simulink model "ClimaRateLimMgr"  (R2022b)
settings: Solver=FixedStepDiscrete  StartTime=0.0  StopTime=10.0  FixedStep=0.2  RelTol=1e-3
(part 1 of 2)
settings Model: Creator=blue.ge  LastModifiedBy=blue.ge
settings DataIOCC: SaveFormat=StructureWithTime
settings OptimizationCC: DefaultParameterBehavior=Inlined  EnhancedBackFolding=on  PassReuseOutputArgsAs=Individual arguments  ZeroExternalMemoryAtStartup=off  ZeroInternalMemoryAtStartup=off  OptimizeBlockOrder=speed  DifferentSizesBufferReuse=on  OptimizationCustomize=off  EfficientTunableParamExpr=on
settings DebuggingCC: ParameterTunabilityLossMsg=error
settings ModelReferenceCC: ModelReferenceNumInstancesAllowed=Single
settings SFSimCC: MATLABDynamicMemAlloc=off
settings RTWCC: Description=AUTOSAR  SystemTargetFile=autosar.tlc  GenCodeOnly=on  TemplateMakefile=ert_default_tmf
settings CodeAppCC: ForceParamTrailComments=on  IgnoreCustomStorageClasses=off  OperatorAnnotations=on  SimulinkDataObjDesc=on  SFDataObjDesc=on  InsertBlockDesc=on
settings STFCustomTargetCC: TargetLangStandard=C99 (ISO)  MultiwordLength=256  ParMdlRefBuildCompliant=on  CompOptLevelCompliant=on  IncludeMdlTerminateFcn=off  CombineOutputUpdateFcns=on  SuppressErrorStatus=on  ERTCustomFileBanners=on  SupportNonFinite=off  SupportComplex=off  SupportContinuousTime=off  SupportNonInlinedSFcns=off  RemoveResetFunc=on  MATLABClassNameForMDSCustomization=Simulink.SoftwareTarget.ERTCustomization  AutosarCompliant=on  UseToolchainInfoCompliant=on  LookupTableObjectStructAxisOrder=2,1,3,4,...  LUTObjectStructOrderEvenSpacing=Size,Table,Breakpoints  SystemTargetFile=autosar.tlc
settings ERTTargetCC: TargetLangStandard=C99 (ISO)  ConcurrentExecutionCompliant=off  IncludeMdlTerminateFcn=off  SuppressErrorStatus=on  SupportNonFinite=off  SupportComplex=off  ModelStepFunctionPrototypeControlCompliant=off  CPPClassGenCompliant=off  AutosarCompliant=on  LookupTableObjectStructAxisOrder=2,1,3,4,...  LUTObjectStructOrderEvenSpacing=Size,Table,Breakpoints  InlinedParameterPlacement=NonHierarchical  ERTCustomFileTemplate=example_file_process.tlc

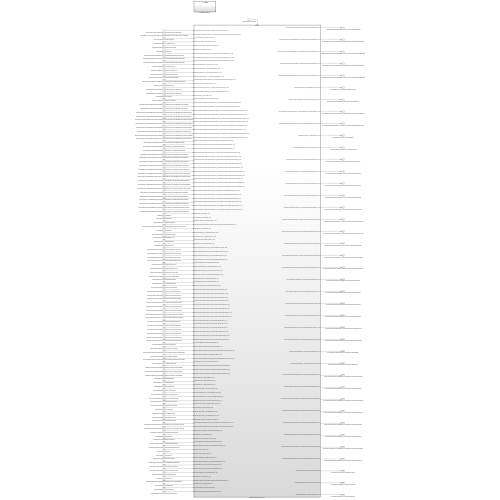
[thumbnail: Simulink's stored preview of the top-level diagram]
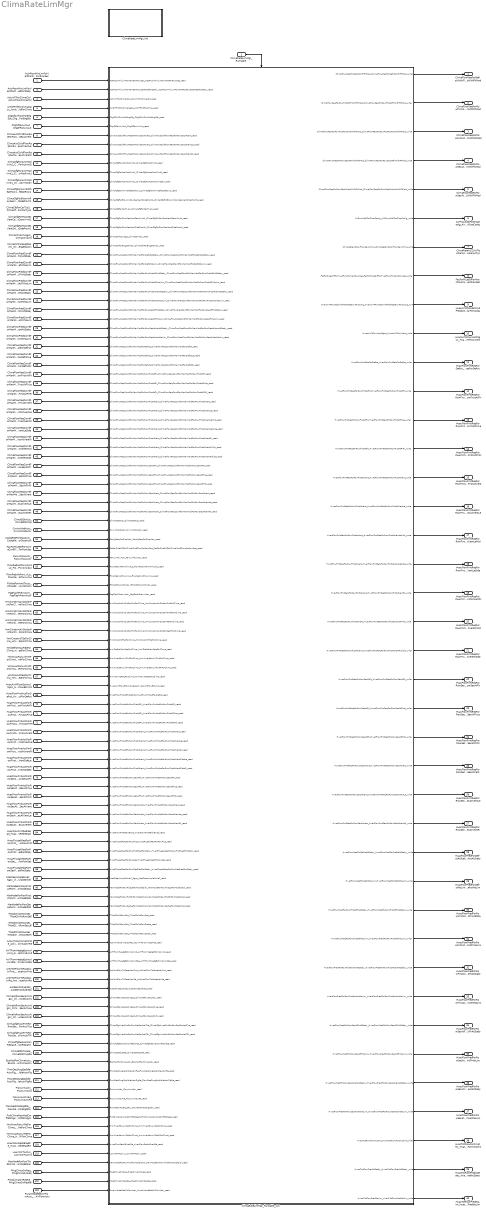
== system ClimaRateLimMgr ==
  ClimaRateLimMgr_Runnable : Inport  SampleTime=0.2  OutputFunctionCall=on
  ActrPosnMinLimForVentForRowSec_ActrPosnMinLimForVentForRowSec : Inport  Port=2  OutDataTypeStr=Perc30  PortDimensions=1
  ActrPosnMinLimForVentForRowSecPredForStaby_ActrPosnMinLimForVentForRowSecPredForStaby : Inport  Port=3  OutDataTypeStr=Perc30  PortDimensions=1
  ActvnTiForClimaCtrl_ActvnTiForClimaCtrl : Inport  Port=4  OutDataTypeStr=Ti33  PortDimensions=1
  AmbTFildForClimaNgcc_AmbTFildForClima : Inport  Port=5  OutDataTypeStr=T30  PortDimensions=1
  ChgOfAirFlowForEngStb_ChgOfAirFlowForEngStb : Inport  Port=6  OutDataTypeStr=Boolean  PortDimensions=1
  ChgOfFanLvlAut_ChgOfFanLvlAut : Inport  Port=7  OutDataTypeStr=Boolean  PortDimensions=1
  ClimaAutCtrldFlowReqForRowSecAtVent_ClimaAutCtrldFlowReqForRowSecAtVent : Inport  Port=8  OutDataTypeStr=AirFlow30  PortDimensions=1
  ClimaAutCtrldFlowReqForRowSecAtVent_ClimaAutCtrldFlowReqForRowSecAtVentLe : Inport  Port=9  OutDataTypeStr=AirFlow30  PortDimensions=1
  ClimaAutCtrldFlowReqForRowSecAtVent_ClimaAutCtrldFlowReqForRowSecAtVentRi : Inport  Port=10  OutDataTypeStr=AirFlow30  PortDimensions=1
  ClimaCfgForActrModVrnt1_ClimaCfgForActrInd1 : Inport  Port=11  OutDataTypeStr=Enum: ClimaCfgForActrInd1  PortDimensions=1
  ClimaCfgForActrModVrnt1_ClimaCfgForActrModVrnt1 : Inport  Port=12  OutDataTypeStr=Enum: ClimaCfgForActrModVrnt1  PortDimensions=1
  ClimaCfgForActrModVrnt1_ClimaCfgForActrModVrntCen : Inport  Port=13  OutDataTypeStr=Enum: ClimaCfgForActrModVrntCen  PortDimensions=1
  ClimaCfgForAmntOfSeatRow1_ClimaCfgForAmntOfSeatRow1 : Inport  Port=14  OutDataTypeStr=Enum: ClimaCfgForAmntOfSeatRow1  PortDimensions=1
  ClimaCfgForBlowrInRowSecVentSidePrsnt1_ClimaCfgForBlowrInRowSecVentSidePrsnt1 : Inport  Port=15  OutDataTypeStr=Boolean  PortDimensions=1
  ClimaCfgForCarTyp1_ClimaCfgForCarTyp1 : Inport  Port=16  OutDataTypeStr=Enum: ClimaCfgForCarTyp1  PortDimensions=1
  ClimaCfgForRowSecVentCentrVrnt1_ClimaCfgForRowSecVentCentrVrnt1 : Inport  Port=17  OutDataTypeStr=Enum: ClimaCfgForRowSecVentCentrVrnt1  PortDimensions=1
  ClimaCfgForRowSecVentSidePrsnt1_ClimaCfgForRowSecVentSidePrsnt1 : Inport  Port=18  OutDataTypeStr=Boolean  PortDimensions=1
  ClimaCtrlActvNgcc_ClimaCtrlActv : Inport  Port=19  OutDataTypeStr=Boolean  PortDimensions=1
  ClimaCtrlForEngStbActv_ClimaCtrlForEngStbActv : Inport  Port=20  OutDataTypeStr=Boolean  PortDimensions=1
  ClimaFlowPredCorrdForManModForDefrstAtStaby_ClimaFlowPredCorrdForManModForDefrstAtStaby : Inport  Port=21  OutDataTypeStr=AirFlow30  PortDimensions=1
  ClimaFlowPredCorrdForManModForDefrstNoLim_ClimaFlowPredCorrdForManModForDefrstNoLim : Inport  Port=22  OutDataTypeStr=AirFlow30  PortDimensions=1
  ClimaFlowPredCorrdForManModForRowFirstAtFlrAtStaby_ClimaFlowPredCorrdForManModForRowFirstAtFlrAtStaby : Inport  Port=23  OutDataTypeStr=AirFlow30  PortDimensions=1
  ClimaFlowPredCorrdForManModForRowFirstAtFlrNoLim_ClimaFlowPredCorrdForManModForRowFirstAtFlrNoLim : Inport  Port=24  OutDataTypeStr=AirFlow30  PortDimensions=1
  ClimaFlowPredCorrdForManModForRowFirstAtVentAtStaby_ClimaFlowPredCorrdForManModForRowFirstAtVentAtStaby : Inport  Port=25  OutDataTypeStr=AirFlow30  PortDimensions=1
  ClimaFlowPredCorrdForManModForRowFirstAtVentNoLim_ClimaFlowPredCorrdForManModForRowFirstAtVentNoLim : Inport  Port=26  OutDataTypeStr=AirFlow30  PortDimensions=1
  ClimaFlowPredCorrdForManModForRowSecAtFlrAtStaby_ClimaFlowPredCorrdForManModForRowSecAtFlrAtStaby : Inport  Port=27  OutDataTypeStr=AirFlow30  PortDimensions=1
  ClimaFlowPredCorrdForManModForRowSecAtFlrNoLim_ClimaFlowPredCorrdForManModForRowSecAtFlrNoLim : Inport  Port=28  OutDataTypeStr=AirFlow30  PortDimensions=1
  ClimaFlowPredCorrdForManModForRowSecAtVentAtStaby_ClimaFlowPredCorrdForManModForRowSecAtVentAtStaby : Inport  Port=29  OutDataTypeStr=AirFlow30  PortDimensions=1
  ClimaFlowPredCorrdForManModForRowSecAtVentNoLim_ClimaFlowPredCorrdForManModForRowSecAtVentNoLim : Inport  Port=30  OutDataTypeStr=AirFlow30  PortDimensions=1
  ClimaFlowReqCorrdForManModForDefrst_ClimaFlowReqCorrdForManModForDefrst : Inport  Port=31  OutDataTypeStr=AirFlow30  PortDimensions=1
  ClimaFlowReqCorrdForManModForDefrst_ClimaFlowReqCorrdForManModForDefrstLe : Inport  Port=32  OutDataTypeStr=AirFlow30  PortDimensions=1
  ClimaFlowReqCorrdForManModForDefrst_ClimaFlowReqCorrdForManModForDefrstRi : Inport  Port=33  OutDataTypeStr=AirFlow30  PortDimensions=1
  ClimaFlowReqCorrdForManModForRowFirstAtFlr_ClimaFlowReqCorrdForManModForRowFirstAtFlr : Inport  Port=34  OutDataTypeStr=AirFlow30  PortDimensions=1
  ClimaFlowReqCorrdForManModForRowFirstAtFlr_ClimaFlowReqCorrdForManModForRowFirstAtFlrLe : Inport  Port=35  OutDataTypeStr=AirFlow30  PortDimensions=1
  ClimaFlowReqCorrdForManModForRowFirstAtFlr_ClimaFlowReqCorrdForManModForRowFirstAtFlrRi : Inport  Port=36  OutDataTypeStr=AirFlow30  PortDimensions=1
  ClimaFlowReqCorrdForManModForRowFirstAtVent_ClimaFlowReqCorrdForManModForRowFirstAtVent : Inport  Port=37  OutDataTypeStr=AirFlow30  PortDimensions=1
  ClimaFlowReqCorrdForManModForRowFirstAtVent_ClimaFlowReqCorrdForManModForRowFirstAtVentLe : Inport  Port=38  OutDataTypeStr=AirFlow30  PortDimensions=1
  ClimaFlowReqCorrdForManModForRowFirstAtVent_ClimaFlowReqCorrdForManModForRowFirstAtVentLeMid : Inport  Port=39  OutDataTypeStr=AirFlow30  PortDimensions=1
  ClimaFlowReqCorrdForManModForRowFirstAtVent_ClimaFlowReqCorrdForManModForRowFirstAtVentLeSide : Inport  Port=40  OutDataTypeStr=AirFlow30  PortDimensions=1
  ClimaFlowReqCorrdForManModForRowFirstAtVent_ClimaFlowReqCorrdForManModForRowFirstAtVentRi : Inport  Port=41  OutDataTypeStr=AirFlow30  PortDimensions=1
  ClimaFlowReqCorrdForManModForRowFirstAtVent_ClimaFlowReqCorrdForManModForRowFirstAtVentRiMid : Inport  Port=42  OutDataTypeStr=AirFlow30  PortDimensions=1
  ClimaFlowReqCorrdForManModForRowFirstAtVent_ClimaFlowReqCorrdForManModForRowFirstAtVentRiSide : Inport  Port=43  OutDataTypeStr=AirFlow30  PortDimensions=1
  ClimaFlowReqCorrdForManModForRowSecAtFlr_ClimaFlowReqCorrdForManModForRowSecAtFlr : Inport  Port=44  OutDataTypeStr=AirFlow30  PortDimensions=1
  ClimaFlowReqCorrdForManModForRowSecAtFlr_ClimaFlowReqCorrdForManModForRowSecAtFlrLe : Inport  Port=45  OutDataTypeStr=AirFlow30  PortDimensions=1
  ClimaFlowReqCorrdForManModForRowSecAtFlr_ClimaFlowReqCorrdForManModForRowSecAtFlrRi : Inport  Port=46  OutDataTypeStr=AirFlow30  PortDimensions=1
  ClimaFlowReqCorrdForManModForRowSecAtVent_ClimaFlowReqCorrdForManModForRowSecAtVent : Inport  Port=47  OutDataTypeStr=AirFlow30  PortDimensions=1
  ClimaFlowReqCorrdForManModForRowSecAtVent_ClimaFlowReqCorrdForManModForRowSecAtVentLe : Inport  Port=48  OutDataTypeStr=AirFlow30  PortDimensions=1
  ClimaFlowReqCorrdForManModForRowSecAtVent_ClimaFlowReqCorrdForManModForRowSecAtVentRi : Inport  Port=49  OutDataTypeStr=AirFlow30  PortDimensions=1
  ClimaStStrtUp_ClimaStStrtUp : Inport  Port=50  OutDataTypeStr=Enum: ClimaStStrtUp  PortDimensions=1
  CnvincModActv_CnvincModActv : Inport  Port=51  OutDataTypeStr=Boolean  PortDimensions=1
  CoolgReqForDispActv_CoolgReqForDispActv : Inport  Port=52  OutDataTypeStr=Enum: CoolgReqForDisp  PortDimensions=1
  FacForRiLeDifForClimaCmftFlowForRowSec_FacForRiLeDifForClimaCmftFlowForRowSec : Inport  Port=53  OutDataTypeStr=Fac30  PortDimensions=1
  FanLvlMaxActv_FanLvlMaxActv : Inport  Port=54  OutDataTypeStr=Boolean  PortDimensions=1
  FlowRednAtPwrAllwdLo_FlowRednAtPwrAllwdLo : Inport  Port=55  OutDataTypeStr=Boolean  PortDimensions=1
  FlowRednAtPwrLvlLo_FlowRednAtPwrLvlLo : Inport  Port=56  OutDataTypeStr=Boolean  PortDimensions=1
  FltModForAmbTActv_FltModForAmbTActv : Inport  Port=57  OutDataTypeStr=Boolean  PortDimensions=1
  FogFlashPrevnActv_FogFlashPrevnActv : Inport  Port=58  OutDataTypeStr=Boolean  PortDimensions=1
  HmiCmptmtAirDistbnFrntForClima_HmiCmptmtAirDistbnFrntForClima : Inport  Port=59  OutDataTypeStr=Enum: HmiCmptmtAirDistbnFrnt  PortDimensions=1
  HmiCmptmtAirDistbnFrntForClima_HmiCmptmtAirDistbnFrntRiForClima : Inport  Port=60  OutDataTypeStr=Enum: HmiCmptmtAirDistbnFrnt  PortDimensions=1
  HmiCmptmtAirDistbnFrntForClima_HmiCmptmtAirDistbnReForClima : Inport  Port=61  OutDataTypeStr=Enum: HmiCmptmtAirDistbnFrnt  PortDimensions=1
  HmiCmptmtAirDistbnFrntForClima_HmiCmptmtAirDistbnReRiForClima : Inport  Port=62  OutDataTypeStr=Enum: HmiCmptmtAirDistbnFrnt  PortDimensions=1
  HmiCmptmtTSpForClima_HmiCmptmtTSpForClima : Inport  Port=63  OutDataTypeStr=Bus: HmiCmptmtTSp  PortDimensions=1  BusOutputAsStruct=on
  HmiDefrstMaxReqForClima_HmiDefrstMaxReqForClima : Inport  Port=64  OutDataTypeStr=Enum: ActrReq  PortDimensions=1
  HmiHvacFanLvlFrntForClima_HmiHvacFanLvlFrntForClima : Inport  Port=65  OutDataTypeStr=Enum: HmiHvacFanLvl  PortDimensions=1
  HmiHvacFanLvlFrntForClima_HmiHvacFanLvlFrntRiForClima : Inport  Port=66  OutDataTypeStr=Enum: HmiHvacFanLvl  PortDimensions=1
  HmiMaxACReqForClima_HmiMaxACReqForClima : Inport  Port=67  OutDataTypeStr=Enum: OnOff1  PortDimensions=1
  HvacAirMFlowEstimd_Ngcc_HvacAirMFlowEstimd : Inport  Port=68  OutMin=0  OutMax=1000  OutDataTypeStr=MFlow1  PortDimensions=1
  HvacFlowFrntActForDefrst_HvacFlowFrntActForDefrst : Inport  Port=69  OutDataTypeStr=AirFlow30  PortDimensions=1
  HvacFlowFrntActForRowFirstAtFlr_HvacFlowFrntActForRowFirstAtFlr : Inport  Port=70  OutDataTypeStr=AirFlow30  PortDimensions=1
  HvacFlowFrntActForRowFirstAtFlr_HvacFlowFrntActForRowFirstAtFlrLe : Inport  Port=71  OutDataTypeStr=AirFlow30  PortDimensions=1
  HvacFlowFrntActForRowFirstAtFlr_HvacFlowFrntActForRowFirstAtFlrRi : Inport  Port=72  OutDataTypeStr=AirFlow30  PortDimensions=1
  HvacFlowFrntActForRowFirstAtVent_HvacFlowFrntActForRowFirstAtVent : Inport  Port=73  OutDataTypeStr=AirFlow30  PortDimensions=1
  HvacFlowFrntActForRowFirstAtVent_HvacFlowFrntActForRowFirstAtVentLe : Inport  Port=74  OutDataTypeStr=AirFlow30  PortDimensions=1
  HvacFlowFrntActForRowFirstAtVent_HvacFlowFrntActForRowFirstAtVentRi : Inport  Port=75  OutDataTypeStr=AirFlow30  PortDimensions=1
  HvacFlowFrntActForRowFirstAtVent_HvacFlowFrntActForRowFirstAtVentSideLe : Inport  Port=76  OutDataTypeStr=AirFlow30  PortDimensions=1
  HvacFlowFrntActForRowFirstAtVent_HvacFlowFrntActForRowFirstAtVentSideRi : Inport  Port=77  OutDataTypeStr=AirFlow30  PortDimensions=1
  HvacFlowFrntActForRowSecAtFlr_HvacFlowFrntActForRowSecAtFlr : Inport  Port=78  OutDataTypeStr=AirFlow30  PortDimensions=1
  HvacFlowFrntActForRowSecAtFlr_HvacFlowFrntActForRowSecAtFlrLe : Inport  Port=79  OutDataTypeStr=AirFlow30  PortDimensions=1
  HvacFlowFrntActForRowSecAtFlr_HvacFlowFrntActForRowSecAtFlrRi : Inport  Port=80  OutDataTypeStr=AirFlow30  PortDimensions=1
  HvacFlowFrntActForRowSecAtVent_HvacFlowFrntActForRowSecAtVent : Inport  Port=81  OutDataTypeStr=AirFlow30  PortDimensions=1
  HvacFlowFrntActForRowSecAtVent_HvacFlowFrntActForRowSecAtVentLe : Inport  Port=82  OutDataTypeStr=AirFlow30  PortDimensions=1
  HvacFlowFrntActForRowSecAtVent_HvacFlowFrntActForRowSecAtVentRi : Inport  Port=83  OutDataTypeStr=AirFlow30  PortDimensions=1
  HvacFlowFrntRednEnad_HvacFlowFrntRednEnad : Inport  Port=84  OutDataTypeStr=Boolean  PortDimensions=1
  HvacFlrLeakReqForRowFirst_HvacFlrLeakReqForRowFirst : Inport  Port=85  OutDataTypeStr=Boolean  PortDimensions=1
  HvacFlrLeakReqForRowFirstPredForStaby_HvacFlrLeakReqForRowFirstPredForStaby : Inport  Port=86  OutDataTypeStr=Boolean  PortDimensions=1
  HvacFlrLeakReqForRowSec_HvacFlrLeakReqForRowSec : Inport  Port=87  OutDataTypeStr=Boolean  PortDimensions=1
  HvacFlrLeakReqForRowSecPredForStaby_HvacFlrLeakReqForRowSecPredForStaby : Inport  Port=88  OutDataTypeStr=Boolean  PortDimensions=1
  InteCleanUnpleSmell_Ngcc_InteCleanUnpleSmell : Inport  Port=89  OutDataTypeStr=Enum: OnOff1  PortDimensions=1
  ManModeForRowFirstLeForModelDial_ManModeForRowFirstLeForModelDial : Inport  Port=90  OutDataTypeStr=Enum: HmiCmptmtAirDistbnFrnt  PortDimensions=1
  ManModeForRowFirstRiForModelDial_ManModeForRowFirstRiForModelDial : Inport  Port=91  OutDataTypeStr=Enum: HmiCmptmtAirDistbnFrnt  PortDimensions=1
  ManModeForRowSecLeForModelDial_ManModeForRowSecLeForModelDial : Inport  Port=92  OutDataTypeStr=Enum: HmiCmptmtAirDistbnFrnt  PortDimensions=1
  TModCtrlAtRowSec_TModCtrlAtRowSec : Inport  Port=93  OutDataTypeStr=T31  PortDimensions=1
  TModCtrlAtRowSec_TModCtrlAtRowSecLe : Inport  Port=94  OutDataTypeStr=T31  PortDimensions=1
  TModCtrlAtRowSec_TModCtrlAtRowSecRi : Inport  Port=95  OutDataTypeStr=T31  PortDimensions=1
  ActvnTiForClimaCtrlRe_ActvnTiForClimaCtrlRe : Inport  Port=96  OutDataTypeStr=Ti33  PortDimensions=1
  AirTFromHeatgEstimdLimd_AirTFromHeatgEstimdLimd : Inport  Port=97  OutDataTypeStr=VCCT30  PortDimensions=1
  AirTFromHeatgEstimdLimdRe_AirTFromHeatgEstimdLimdRe : Inport  Port=98  OutDataTypeStr=VCCT30  PortDimensions=1
  AntiHotFlowToFaceActvFrnt_AntiHotFlowToFaceActvFrnt : Inport  Port=99  OutDataTypeStr=Boolean  PortDimensions=1
  AntiHotFlowToFaceActvRe_AntiHotFlowToFaceActvRe : Inport  Port=100  OutDataTypeStr=Boolean  PortDimensions=1
  AutDemstOperSts_AutDemstOperSts : Inport  Port=101  OutDataTypeStr=Enum: OnOff1  PortDimensions=1
  ClimaAtRowSecActvNgcc_ClimaAtRowSecActv : Inport  Port=102  OutDataTypeStr=Boolean  PortDimensions=1
  ClimaAtRowSecActvNgcc_ClimaAtRowSecActvLe : Inport  Port=103  OutDataTypeStr=Boolean  PortDimensions=1
  ClimaAtRowSecActvNgcc_ClimaAtRowSecActvRi : Inport  Port=104  OutDataTypeStr=Boolean  PortDimensions=1
  ClimaCfgHvacFrntForRowSecForActrTLe_ClimaCfgHvacFrntForRowSecForActrTLe : Inport  Port=105  OutDataTypeStr=Boolean  PortDimensions=1
  ClimaCfgHvacFrntForRowSecForActrTRi_ClimaCfgHvacFrntForRowSecForActrTRi : Inport  Port=106  OutDataTypeStr=Boolean  PortDimensions=1
  ClimaCfgReqVersionReplace_ClimaCfgReqVersionReplace : Inport  Port=107  OutDataTypeStr=UInt8  PortDimensions=1
  ClimaStStrtUpRe_ClimaStStrtUpRe : Inport  Port=108  OutDataTypeStr=Enum: ClimaStStrtUp  PortDimensions=1
  EcoModForClimaActv_EcoModForClimaActv : Inport  Port=109  OutDataTypeStr=Enum: OnOff1  PortDimensions=1
  FlowDecDurgSpdIdleActvFlg_FlowDecDurgSpdIdleActvFlg : Inport  Port=110  OutDataTypeStr=Boolean  PortDimensions=1
  FlowDecDurgSpdIdleActvFlgRe_FlowDecDurgSpdIdleActvFlgRe : Inport  Port=111  OutDataTypeStr=Boolean  PortDimensions=1
  FlowLimActv_FlowLimActv : Inport  Port=112  OutDataTypeStr=Boolean  PortDimensions=1
  FlowLimActvRe_FlowLimActvRe : Inport  Port=113  OutDataTypeStr=Boolean  PortDimensions=1
  FlowRednForEngStby_FlowRednForEngStby : Inport  Port=114  OutDataTypeStr=Boolean  PortDimensions=1
  FrntClimaManModCmftStsNgcc_FrntClimaManModCmftStsNgcc : Inport  Port=115  OutDataTypeStr=Enum: ClimaManModCmftSts  PortDimensions=1
  HmiHvacFanLvlReForClima_HmiHvacFanLvlReForClima : Inport  Port=116  OutDataTypeStr=Enum: HmiHvacFanLvl  PortDimensions=1
  HmiHvacFanLvlReForClima_HmiHvacFanLvlReRiForClima : Inport  Port=117  OutDataTypeStr=Enum: HmiHvacFanLvl  PortDimensions=1
  HvacFlowRednEnadRe_HvacFlowRednEnadRe : Inport  Port=118  OutDataTypeStr=Boolean  PortDimensions=1
  LowrWtrTActvn_LowrWtrTActvn : Inport  Port=119  OutDataTypeStr=Boolean  PortDimensions=1
  ManModeForRowThrdForModelDial_ManModeForRowThrdForModelDial : Inport  Port=120  OutDataTypeStr=Enum: HmiCmptmtAirDistbnFrnt  PortDimensions=1
  PrkgClimaOnReq_PrkgClimaOnReq : Inport  Port=121  OutDataTypeStr=Boolean  PortDimensions=1
  PrkgClimaOnReqRe_PrkgClimaOnReqRe : Inport  Port=122  OutDataTypeStr=Enum: OnOff1  PortDimensions=1
  RuranModeSetAirFlowActv_RuranModeSetAirFlowActv : Inport  Port=123  OutDataTypeStr=Boolean  PortDimensions=1
  ClimaRateLimMgr_Init : Subsystem
  ClimaRateLimMgr_Runnable_sys : Subsystem
  ClimaFlowReqForDefrstWithFltMod_ClimaFlowReqForDefrstWithFltMod : Outport  OutDataTypeStr=AirFlow30  PortDimensions=1
  ClimaFlowReqForRowFirstAtFlrWithFltMod_ClimaFlowReqForRowFirstAtFlrWithFltMod : Outport  Port=2  OutDataTypeStr=AirFlow30  PortDimensions=1
  ClimaFlowReqForRowFirstAtVentWithFltMod_ClimaFlowReqForRowFirstAtVentWithFltMod : Outport  Port=3  OutDataTypeStr=AirFlow30  PortDimensions=1
  ClimaFlowReqForRowSecAtFlrWithFltMod_ClimaFlowReqForRowSecAtFlrWithFltMod : Outport  Port=4  OutDataTypeStr=AirFlow30  PortDimensions=1
  ClimaFlowReqForRowSecAtVentWithFltMod_ClimaFlowReqForRowSecAtVentWithFltMod : Outport  Port=5  OutDataTypeStr=AirFlow30  PortDimensions=1
  AirFlowAddlForDispCoolg_AirFlowAddlForDispCoolg : Outport  Port=6  OutDataTypeStr=AirFlow30  PortDimensions=1
  ClimaRateVCCAirFlowRampTyp_ClimaRateVCCAirFlowRampTyp : Outport  Port=7  OutDataTypeStr=Enum: VCCAirFlowRampTyp  PortDimensions=1
  FacForRiLeDifForHvacFlowForVentRowSec_FacForRiLeDifForHvacFlowForVentRowSec : Outport  Port=8  OutDataTypeStr=Fac30  PortDimensions=1
  HvacAirMFlowEstimdPredAtStabyForCoolg_HvacAirMFlowEstimdPredAtStabyForCoolg : Outport  Port=9  OutDataTypeStr=AirFlow30  PortDimensions=1
  HvacAirMFlowVentNgcc_HvacAirMFlowVent : Outport  Port=10  OutMin=0  OutMax=1000  OutDataTypeStr=MFlow1  PortDimensions=1
  HvacFlowFrntReqForDefrst_HvacFlowFrntReqForDefrst : Outport  Port=11  OutDataTypeStr=AirFlow30  PortDimensions=1
  HvacFlowFrntReqForRowFirstAtFlr_HvacFlowFrntReqForRowFirstAtFlr : Outport  Port=12  OutDataTypeStr=AirFlow30  PortDimensions=1
  HvacFlowFrntReqForRowFirstAtFlr_HvacFlowFrntReqForRowFirstAtFlrLe : Outport  Port=13  OutDataTypeStr=AirFlow30  PortDimensions=1
  HvacFlowFrntReqForRowFirstAtFlr_HvacFlowFrntReqForRowFirstAtFlrRi : Outport  Port=14  OutDataTypeStr=AirFlow30  PortDimensions=1
  HvacFlowFrntReqForRowFirstAtVent_HvacFlowFrntReqForRowFirstAtVent : Outport  Port=15  OutDataTypeStr=AirFlow30  PortDimensions=1
  HvacFlowFrntReqForRowFirstAtVent_HvacFlowFrntReqForRowFirstAtVentLe : Outport  Port=16  OutDataTypeStr=AirFlow30  PortDimensions=1
  HvacFlowFrntReqForRowFirstAtVent_HvacFlowFrntReqForRowFirstAtVentLeMid : Outport  Port=17  OutDataTypeStr=AirFlow30  PortDimensions=1
  HvacFlowFrntReqForRowFirstAtVent_HvacFlowFrntReqForRowFirstAtVentLeSide : Outport  Port=18  OutDataTypeStr=AirFlow30  PortDimensions=1
  HvacFlowFrntReqForRowFirstAtVent_HvacFlowFrntReqForRowFirstAtVentRi : Outport  Port=19  OutDataTypeStr=AirFlow30  PortDimensions=1
  HvacFlowFrntReqForRowFirstAtVent_HvacFlowFrntReqForRowFirstAtVentRiMid : Outport  Port=20  OutDataTypeStr=AirFlow30  PortDimensions=1
  HvacFlowFrntReqForRowFirstAtVent_HvacFlowFrntReqForRowFirstAtVentRiSide : Outport  Port=21  OutDataTypeStr=AirFlow30  PortDimensions=1
  HvacFlowFrntReqForRowSecAtFlr_HvacFlowFrntReqForRowSecAtFlr : Outport  Port=22  OutDataTypeStr=AirFlow30  PortDimensions=1
  HvacFlowFrntReqForRowSecAtFlr_HvacFlowFrntReqForRowSecAtFlrLe : Outport  Port=23  OutDataTypeStr=AirFlow30  PortDimensions=1
  HvacFlowFrntReqForRowSecAtFlr_HvacFlowFrntReqForRowSecAtFlrRi : Outport  Port=24  OutDataTypeStr=AirFlow30  PortDimensions=1
  HvacFlowFrntReqForRowSecAtVent_HvacFlowFrntReqForRowSecAtVent : Outport  Port=25  OutDataTypeStr=AirFlow30  PortDimensions=1
  HvacFlowFrntReqForRowSecAtVent_HvacFlowFrntReqForRowSecAtVentLe : Outport  Port=26  OutDataTypeStr=AirFlow30  PortDimensions=1
  HvacFlowFrntReqForRowSecAtVent_HvacFlowFrntReqForRowSecAtVentRi : Outport  Port=27  OutDataTypeStr=AirFlow30  PortDimensions=1
  HvacFlowPredForDefrstAtStaby_HvacFlowPredForDefrstAtStaby : Outport  Port=28  OutDataTypeStr=AirFlow30  PortDimensions=1
  HvacFlowPredForDefrstNoLim_HvacFlowPredForDefrstNoLim : Outport  Port=29  OutDataTypeStr=AirFlow30  PortDimensions=1
  HvacFlowPredForRowFirstAtFlrAtStaby_HvacFlowPredForRowFirstAtFlrAtStaby : Outport  Port=30  OutDataTypeStr=AirFlow30  PortDimensions=1
  HvacFlowPredForRowFirstAtFlrNoLim_HvacFlowPredForRowFirstAtFlrNoLim : Outport  Port=31  OutDataTypeStr=AirFlow30  PortDimensions=1
  HvacFlowPredForRowFirstAtVentAtStaby_HvacFlowPredForRowFirstAtVentAtStaby : Outport  Port=32  OutDataTypeStr=AirFlow30  PortDimensions=1
  HvacFlowPredForRowFirstAtVentNoLim_HvacFlowPredForRowFirstAtVentNoLim : Outport  Port=33  OutDataTypeStr=AirFlow30  PortDimensions=1
  HvacFlowPredForRowSecAtFlrAtStaby_HvacFlowPredForRowSecAtFlrAtStaby : Outport  Port=34  OutDataTypeStr=AirFlow30  PortDimensions=1
  HvacFlowPredForRowSecAtFlrNoLim_HvacFlowPredForRowSecAtFlrNoLim : Outport  Port=35  OutDataTypeStr=AirFlow30  PortDimensions=1
  HvacFlowPredForRowSecAtVentAtStaby_HvacFlowPredForRowSecAtVentAtStaby : Outport  Port=36  OutDataTypeStr=AirFlow30  PortDimensions=1
  HvacFlowPredForRowSecAtVentNoLim_HvacFlowPredForRowSecAtVentNoLim : Outport  Port=37  OutDataTypeStr=AirFlow30  PortDimensions=1
  HvacTotFlowForCmptmt_HvacTotFlowForCmptmt : Outport  Port=38  OutDataTypeStr=AirFlow30  PortDimensions=1
  HvacTotFlowPredAtStaby_HvacTotFlowPredAtStaby : Outport  Port=39  OutDataTypeStr=AirFlow30  PortDimensions=1
  HvacTotFlowPredNoLim_HvacTotFlowPredNoLim : Outport  Port=40  OutDataTypeStr=AirFlow30  PortDimensions=1
  wires:
  ActrPosnMinLimForVentForRowSec_ActrPosnMinLimForVentForRowSec/1 -> ClimaRateLimMgr_Runnable_sys/1(ActrPosnMinLimForVentForRowSec_ActrPosnMinLimForVentForRowSec_read)
  ActrPosnMinLimForVentForRowSecPredForStaby_ActrPosnMinLimForVentForRowSecPredForStaby/1 -> ClimaRateLimMgr_Runnable_sys/2(ActrPosnMinLimForVentForRowSecPredForStaby_ActrPosnMinLimForVentForRowSecPredForStaby_read)
  ActvnTiForClimaCtrl_ActvnTiForClimaCtrl/1 -> ClimaRateLimMgr_Runnable_sys/3(ActvnTiForClimaCtrl_ActvnTiForClimaCtrl_read)
  AmbTFildForClimaNgcc_AmbTFildForClima/1 -> ClimaRateLimMgr_Runnable_sys/4(AmbTFildForClimaNgcc_AmbTFildForClima_read)
  ChgOfAirFlowForEngStb_ChgOfAirFlowForEngStb/1 -> ClimaRateLimMgr_Runnable_sys/5(ChgOfAirFlowForEngStb_ChgOfAirFlowForEngStb_read)
  ChgOfFanLvlAut_ChgOfFanLvlAut/1 -> ClimaRateLimMgr_Runnable_sys/6(ChgOfFanLvlAut_ChgOfFanLvlAut_read)
  ClimaAutCtrldFlowReqForRowSecAtVent_ClimaAutCtrldFlowReqForRowSecAtVent/1 -> ClimaRateLimMgr_Runnable_sys/7(ClimaAutCtrldFlowReqForRowSecAtVent_ClimaAutCtrldFlowReqForRowSecAtVent_read)
  ClimaAutCtrldFlowReqForRowSecAtVent_ClimaAutCtrldFlowReqForRowSecAtVentLe/1 -> ClimaRateLimMgr_Runnable_sys/8(ClimaAutCtrldFlowReqForRowSecAtVent_ClimaAutCtrldFlowReqForRowSecAtVentLe_read)
  ClimaAutCtrldFlowReqForRowSecAtVent_ClimaAutCtrldFlowReqForRowSecAtVentRi/1 -> ClimaRateLimMgr_Runnable_sys/9(ClimaAutCtrldFlowReqForRowSecAtVent_ClimaAutCtrldFlowReqForRowSecAtVentRi_read)